simulink model "ofdm_txrx_mimo"  (Simulink 7.1)
settings: Solver=ode45  StartTime=0.0  StopTime=6e3  RelTol=1e-3
PreLoadFcn:
  | ofdm_rx_mimo_init
InitFcn:
  | ofdm_rx_mimo_init
StartFcn:
  | ofdm_rx_mimo_init
values from neighbouring files (symbols this model uses):
  BER_RAM_init_size = 2048    [ofdm_tx_mimo_init.m]
  CPLength = 16    [ofdm_tx_mimo_init.m]
  CRCPolynomial = hex2dec('04c11db7')    [ofdm_tx_mimo_init.m]
  freqOffset_track_filtCoef_i = hex2dec('2500')/2^32    [ofdm_rx_mimo_init.m]
  freqOffset_track_filtCoef_p = hex2dec('d0000')/2^32    [ofdm_rx_mimo_init.m]
  hi = zeros(1,length(cci))    [ofdm_rx_mimo_init.m]
  hr = zeros(1,length(ccr))    [ofdm_rx_mimo_init.m]
  max_CP_length = 16    [ofdm_tx_mimo_init.m]
  max_num_baseRateSymbols = 31    [ofdm_tx_mimo_init.m]
  max_num_subcarriers = 64    [ofdm_tx_mimo_init.m]
  max_num_trainingSymbols = 15    [ofdm_tx_mimo_init.m]
  max_numBytes = 16384    [ofdm_tx_mimo_init.m]
  max_OFDM_symbols = 2047    [ofdm_tx_mimo_init.m]
  modConstellation_qam16 = .75*[1 3 -1 -3]./3    [ofdm_tx_mimo_init.m]
  modConstellation_qam256 = 0.9375*[3 1 5 7 11 9 13 15 -3 -1 -5 -7 -11 -9 -13 -15]./15    [ofdm_tx_mimo_init.m]
  modConstellation_qam64 = 0.875*[3 1 5 7 -3 -1 -5 -7]./7    [ofdm_tx_mimo_init.m]
  modConstellation_qpsk = [1 -1]./sqrt(2)    [ofdm_tx_mimo_init.m]
  numBaseRateSymbols = 2    [ofdm_tx_mimo_init.m]
  numSubcarriers = 64    [ofdm_tx_mimo_init.m]
  numTrainingSymbols = 2    [ofdm_tx_mimo_init.m]
  pilot1_indicies = 7 + ( (64-7) * 2^16)    (= 3735559)    [ofdm_tx_mimo_init.m]
  pilot2_indicies = 21 + ( (64-21) * 2^16)    (= 2818069)    [ofdm_tx_mimo_init.m]
  pilotValue_neg = hex2dec('8000') + (2^16 * 0)    [ofdm_tx_mimo_init.m]
  pilotValue_pos = hex2dec('7FFF') + (2^16 * 0)    [ofdm_tx_mimo_init.m]
  pkt_crossCorr_thresh = 0.7    [ofdm_rx_mimo_init.m]
  pkt_energy_thresh = 0    [ofdm_rx_mimo_init.m]
  pkt_numPayloadBytes = 1400+42-6    (= 1436)    [ofdm_tx_mimo_init.m]
  pktByteNums = numHeaderBytes +  				byteIndex_numPayloadBytes(1)*2^8 +  				byteIndex_numPayloadBytes(2)*2^16 +  				byteIndex_simpleDynModMasks * 2^24    [ofdm_rx_mimo_init.m]
  PktDet_Delay = 26 + Symbol_Timing_Offset*2^7    (= 1050)    [ofdm_rx_mimo_init.m]
  preamble = 6*[0 shortsyms_10 longsyms_2]    [ofdm_tx_mimo_init.m]
  preamble_I = real(preamble)    [ofdm_tx_mimo_init.m]
  preamble_Q = imag(preamble)    [ofdm_tx_mimo_init.m]
  RAM_init_size = max_numBytes    (= 16384)    [ofdm_tx_mimo_init.m]
  reg_Interrupt_PktBuf_Control = reg_InterruptControl +  	1 * 2^16 +  	0 * 2^24    (= 65656)    [ofdm_rx_mimo_init.m]
  rx_controlBits = 1 * 2  		 + 1 * 4  		 + 0 * 8  		 + 1 * 16 * rx_SISO_Mode  		 + 1 * 32  		 + 1 * 64  		 + 0 * 128  		 + 1 * 256  		 + 0 * 512  		 + 1 * 1024  		 + 0 * 2048  		 + 1 * 4096  		 + 1 * 8192  		 + 0 * 16384  		 + 1 * 65536  		 + 1 * 131072  		 + 0 * 262144  		 + 1 * 524288    [ofdm_rx_mimo_init.m]
  Rx_PhaseNoiseTrack_K = 0.6094    [ofdm_rx_mimo_init.m]
  rx_postEq_scaling = round(rxScaling*2^11) * (1 + 2^16)    [ofdm_rx_mimo_init.m]
  RxDataScrambling_Seq = [40 198 78 63 82 173 102 245 48 111 172 115 147 230 216 93 72 65 62 2 205 242 122 90 128 83 105 97 73 10 5 252]    [ofdm_tx_mimo_init.m]
  simOnly_numSamples = length(preamble)+( (numSubcarriers+CPLength)*(numTrainingSymbols + numBaseRateSymbols + numFullRateSymbols/2) )    [ofdm_tx_mimo_init.m]
  train = [train train]    [ofdm_tx_mimo_init.m]
  tx_controlBits = (antB_preambleShift * 2^4) + (2^0 * tx_SISO_Mode) + 4*0 + 8*1    (= 73)    [ofdm_tx_mimo_init.m]
  TxDataScrambling_Seq = [40 198 78 63 82 173 102 245 48 111 172 115 147 230 216 93 72 65 62 2 205 242 122 90 128 83 105 97 73 10 5 252]    [ofdm_tx_mimo_init.m]
  txReg_symbolCounts = (2^16 * numFullRateSymbols) + (2^8 *numBaseRateSymbols ) + numTrainingSymbols    [ofdm_tx_mimo_init.m]
  TxRx_FFTScaling = bin2dec('010111') + (bin2dec('000101') * 2^6)    [ofdm_tx_mimo_init.m]
undefined symbols (not defined in the file or in its folder): AntA, AntB, BaseRate, CRC_table_gen, gcbh, intfilt, pktTiming_count_payloadStart, pktTiming_symbol_length, xlBlockLoadCallback, xlGetSimulinkPeriod
libraries: xbsIndex_r4 x4212, dspmlti4 x4, dspsigops x4, commchan3 x2, simulink x2, commrflib2 x2, dspsrcs4 x1
(part 15 of 43)

== system ofdm_txrx_mimo/OFDM Tx MIMO/TxControl/FFT Start Gen ==
  note: First two symbols are always training and should be processed immediately, back-to-back. The same IFFT
      | output will be repeated numTrainingSyms times. After this, the IFFT processes the base-rate symbols. Because
      | each base-rate symbol is transmitted from both antennas, the IFFT waits a full output symbol period before processing
      | the next symbol. After the base rate symbols, the multiplexing of full-rate symbols begins, so the IFFT is triggered twice
      | per output symbol period (computing two output symbols- one per antenna- per period).
  SymNum : Inport  OutDataType=sfix(16)  OutScaling=2^0
  AddSub1 : xbsIndex_r4/AddSub  mode=Addition  use_carryin=off  use_carryout=off  en=off  latency=0  precision=Full  arith_type=Unsigned  n_bits=10  bin_pt=0  quantization=Truncate  overflow=Wrap  dbl_ovrd=off  use_behavioral_HDL=on  pipelined=off  use_rpm=on  hw_selection=Fabric  block_type=addsub
      settings: xl_area=[0, 0, 0, 0, 0, 0, 0]  block_version=VER_STRING_GOES_HERE
  Constant9 : xbsIndex_r4/Constant  arith_type=Unsigned  const=1  n_bits=1  bin_pt=0  explicit_period=off  period=1  equ=P=C  opselect=C  inp2=PCIN>>17  opr=+  inp1=P  carry=CIN  dbl_ovrd=off  block_type=constant
      settings: block_version=VER_STRING_GOES_HERE
  Inverter : xbsIndex_r4/Inverter  en=off  latency=0  dbl_ovrd=off  block_type=inv
      settings: block_version=VER_STRING_GOES_HERE
  LSB : xbsIndex_r4/Slice  nbits=1  boolean_output=on  mode=Lower Bit Location + Width  bit1=0  base1=LSB of Input  bit0=0  base0=LSB of Input  dbl_ovrd=off  block_type=slice
      settings: infoedit="Extracts a given range of bits from each input sample and presents it at the output.  The output type is ordinarily unsigned with binary point at zero, but can be Boolean when the slice is one bit wide.<P><P>Hardware notes: In hardware this block costs nothing."  block_version=VER_STRING_GOES_HERE
  Logical : xbsIndex_r4/Logical  logical_function=OR  inputs=3  en=off  latency=0  precision=Full  arith_type=Unsigned  n_bits=16  bin_pt=0  align_bp=on  dbl_ovrd=off  block_type=logical
      settings: block_version=VER_STRING_GOES_HERE
  Logical1 : xbsIndex_r4/Logical  logical_function=AND  inputs=3  en=off  latency=0  precision=Full  arith_type=Unsigned  n_bits=16  bin_pt=0  align_bp=on  dbl_ovrd=off  block_type=logical
      settings: block_version=VER_STRING_GOES_HERE
  Output Scope2 : Scope  NumInputPorts=6
      settings: TimeRange=2200  YMin=0~0~0~-1~-1~-1  YMax=1~1~1~1~1~1  SaveName=ScopeData7
  Relational1 : xbsIndex_r4/Relational  mode=a>=b  en=off  latency=0  dbl_ovrd=off  block_type=relational
      settings: xl_area=[2 0 0 4 0 0 0]  block_version=VER_STRING_GOES_HERE
  Relational2 : xbsIndex_r4/Relational  mode=a<b  en=off  latency=0  dbl_ovrd=off  block_type=relational
      settings: xl_area=[2 0 0 4 0 0 0]  block_version=VER_STRING_GOES_HERE
  Relational3 : xbsIndex_r4/Relational  mode=a>=b  en=off  latency=0  dbl_ovrd=off  block_type=relational
      settings: xl_area=[2 0 0 4 0 0 0]  block_version=VER_STRING_GOES_HERE
  Relational9 : xbsIndex_r4/Relational  mode=a<=b  en=off  latency=0  dbl_ovrd=off  block_type=relational
      settings: xl_area=[2 0 0 4 0 0 0]  block_version=VER_STRING_GOES_HERE
  En : Outport  OutDataType=sfix(16)  OutScaling=2^0
  wires:
  Constant9/1 -> Relational9/2
  SymNum/1 -> Relational9/1, Relational1/1, Relational2/1, LSB/1, Relational3/1, Output Scope2/1
  Relational9/1 -> Logical/1, Output Scope2/2
  Logical/1 -> En/1, Output Scope2/5
  [reg_numTrainingSyms] -> Relational1/2, AddSub1/1
  Relational1/1 -> Logical1/1
  AddSub1/1 -> Relational2/2, Relational3/2
  [reg_numBaseRateSyms] -> AddSub1/2
  Relational2/1 -> Logical1/2
  LSB/1 -> Inverter/1
  Logical1/1 -> Logical/2, Output Scope2/3
  Relational3/1 -> Logical/3, Output Scope2/4
  Inverter/1 -> Logical1/3

== system ofdm_txrx_mimo/OFDM Tx MIMO/TxControl/Posedge1 ==
  D : Inport  OutDataType=sfix(16)  OutScaling=2^0
  Delay : xbsIndex_r4/Delay  en=off  latency=1  dbl_ovrd=off  reg_retiming=off  block_type=delay
      settings: infoedit=Hardware notes: A delay line is a chain, each link of which is an SRL16 followed by a flip-flop. If register retiming is enabled, the delay line is a chain of flip-flops.  xl_area=[1 1 0 0 0 0 0]  block_version=VER_STRING_GOES_HERE
  Inverter : xbsIndex_r4/Inverter  en=off  latency=0  dbl_ovrd=off  block_type=inv
      settings: xl_area=[0 0 0 0 0 0 0]  block_version=VER_STRING_GOES_HERE
  Logical : xbsIndex_r4/Logical  logical_function=AND  inputs=2  en=off  latency=0  precision=Full  arith_type=Unsigned  n_bits=8  bin_pt=2  align_bp=on  dbl_ovrd=off  block_type=logical
      settings: xl_area=[1 0 0 1 0 0 0]  block_version=VER_STRING_GOES_HERE
  Q : Outport  OutDataType=sfix(16)  OutScaling=2^0
  wires:
  Logical/1 -> Q/1
  D/1 -> Delay/1, Logical/1
  Inverter/1 -> Logical/2
  Delay/1 -> Inverter/1

== system ofdm_txrx_mimo/OFDM Tx MIMO/TxControl/Posedge2 ==
  D : Inport  OutDataType=sfix(16)  OutScaling=2^0
  Delay : xbsIndex_r4/Delay  en=off  latency=1  dbl_ovrd=off  reg_retiming=off  block_type=delay
      settings: infoedit=Hardware notes: A delay line is a chain, each link of which is an SRL16 followed by a flip-flop. If register retiming is enabled, the delay line is a chain of flip-flops.  xl_area=[1 1 0 0 0 0 0]  block_version=VER_STRING_GOES_HERE
  Inverter : xbsIndex_r4/Inverter  en=off  latency=0  dbl_ovrd=off  block_type=inv
      settings: xl_area=[0 0 0 0 0 0 0]  block_version=VER_STRING_GOES_HERE
  Logical : xbsIndex_r4/Logical  logical_function=AND  inputs=2  en=off  latency=0  precision=Full  arith_type=Unsigned  n_bits=1  bin_pt=0  align_bp=on  dbl_ovrd=off  block_type=logical
      settings: xl_area=[1 0 0 1 0 0 0]  block_version=VER_STRING_GOES_HERE
  Q : Outport  OutDataType=sfix(16)  OutScaling=2^0
  wires:
  Delay/1 -> Inverter/1
  Inverter/1 -> Logical/2
  D/1 -> Logical/1, Delay/1
  Logical/1 -> Q/1

== system ofdm_txrx_mimo/OFDM Tx MIMO/TxControl/Start/Done Control ==
  TxDone : Inport  OutDataType=sfix(16)  OutScaling=2^0
  Start : Inport  Port=2  OutDataType=sfix(16)  OutScaling=2^0
  Constant2 : xbsIndex_r4/Constant  arith_type=Boolean  const=1  n_bits=1  bin_pt=0  explicit_period=off  period=1  equ=P=C  opselect=C  inp2=PCIN>>17  opr=+  inp1=P  carry=CIN  dbl_ovrd=off  block_type=constant
      settings: block_version=VER_STRING_GOES_HERE
  Convert1 : xbsIndex_r4/Convert  arith_type=Boolean  n_bits=16  bin_pt=14  quantization=Truncate  overflow=Wrap  latency=0  dbl_ovrd=off  pipeline=off  block_type=convert
      settings: block_version=8.2.02
  Convert2 : xbsIndex_r4/Convert  arith_type=Boolean  n_bits=16  bin_pt=14  quantization=Truncate  overflow=Wrap  latency=0  dbl_ovrd=off  pipeline=off  block_type=convert
      settings: block_version=8.2.02
  Inverter : xbsIndex_r4/Inverter  en=off  latency=0  dbl_ovrd=off  block_type=inv
      settings: block_version=8.2.02
  Logical1 : xbsIndex_r4/Logical  logical_function=OR  inputs=2  en=off  latency=0  precision=Full  arith_type=Unsigned  n_bits=8  bin_pt=2  align_bp=on  dbl_ovrd=off  block_type=logical
      settings: xl_area=[1 0 0 1 0 0 0]  block_version=VER_STRING_GOES_HERE
  Logical4 : xbsIndex_r4/Logical  logical_function=AND  inputs=2  en=off  latency=0  precision=Full  arith_type=Unsigned  n_bits=8  bin_pt=2  align_bp=on  dbl_ovrd=off  block_type=logical
      settings: xl_area=[1 0 0 1 0 0 0]  block_version=VER_STRING_GOES_HERE
  Posedge2 : Subsystem
  Posedge3 : Subsystem
  S-R Latch : Subsystem
  Tx_PktDone : xbsIndex_r4/Gateway Out  hdl_port=on  timing_constraint=None  locs_specified=off  LOCs={}  block_type=gatewayout
      settings: infoedit="Gateway out block.  Converts Xilinx fixed point inputs into ouputs of type Simulink integer, double, or fixed point.<P><P>Hardware notes:  In hardware these blocks become top level output ports or are discarded, depending on how they are configured."  xl_area=[0 0 0 0 1 0 0]  block_version=VER_STRING_GOES_HERE
  Up Sample1 : xbsIndex_r4/Up Sample  sample_ratio=2  copy_samples=on  dbl_ovrd=off  block_type=usamp
      settings: infoedit="Up samples input data.  Inserted values  can be zeros or copies of the most recent input sample.<P><P>Hardware notes: No hardware is needed if inserted values are copies of the input sample; otherwise, a mux and single bit flip-flop are used."  block_version=VER_STRING_GOES_HERE
  Up Sample2 : xbsIndex_r4/Up Sample  sample_ratio=2  copy_samples=on  dbl_ovrd=off  block_type=usamp
      settings: infoedit="Up samples input data.  Inserted values  can be zeros or copies of the most recent input sample.<P><P>Hardware notes: No hardware is needed if inserted values are copies of the input sample; otherwise, a mux and single bit flip-flop are used."  block_version=VER_STRING_GOES_HERE
  TxStart : Outport  OutDataType=sfix(16)  OutScaling=2^0
  tag scope: regTx_PktRunning=global, regTx_PktRunningEn=global
  wires:
  Start/1 -> Posedge2/1(D)
  TxDone/1 -> Posedge3/1(D)
  Logical1/1 -> S-R Latch/2(R)
  [TxReset] -> Logical1/2
  S-R Latch/1(Q) -> Up Sample1/1, Inverter/1
  Constant2/1 -> [regTx_PktRunningEn]
  Up Sample1/1 -> [regTx_PktRunning]
  Inverter/1 -> Convert2/1
  Logical4/1 -> TxStart/1
  Posedge3/1(Q) -> Logical1/1, Up Sample2/1
  Convert1/1 -> Logical4/1
  Convert2/1 -> Logical4/2
  Posedge2/1(Q) -> S-R Latch/1(S), Convert1/1
  Up Sample2/1 -> Tx_PktDone/1

== system ofdm_txrx_mimo/OFDM Tx MIMO/TxStart/Copy_Downsample ==
  D : Inport  OutDataType=sfix(16)  OutScaling=2^0
  Delay : xbsIndex_r4/Delay  en=off  latency=1  dbl_ovrd=off  reg_retiming=off  block_type=delay
      settings: infoedit=Hardware notes: A delay line is a chain, each link of which is an SRL16 followed by a flip-flop. If register retiming is enabled, the delay line is a chain of flip-flops.  block_version=VER_STRING_GOES_HERE
  Down Sample : xbsIndex_r4/Down Sample  sample_ratio=2  sample_phase="Last Value of Frame  (most efficient)"  en=off  latency=1  dbl_ovrd=off  block_type=dsamp
      settings: block_version=VER_STRING_GOES_HERE
  Logical : xbsIndex_r4/Logical  logical_function=OR  inputs=2  en=off  latency=0  precision=Full  arith_type=Unsigned  n_bits=16  bin_pt=0  align_bp=on  dbl_ovrd=off  block_type=logical
      settings: block_version=VER_STRING_GOES_HERE
  Q : Outport  OutDataType=sfix(16)  OutScaling=2^0
  wires:
  Down Sample/1 -> Q/1
  Logical/1 -> Down Sample/1
  D/1 -> Logical/2, Delay/1
  Delay/1 -> Logical/1

== system ofdm_txrx_mimo/Memory-mapped Registers/TxRx Registers/InterruptControl/Posedge ==
  note: Rising Edge Detection
  D : Inport  OutDataType=sfix(16)  OutScaling=2^0
  Inverter : xbsIndex_r4/Inverter  en=off  latency=1  dbl_ovrd=off  block_type=inv
      settings: xl_area=[0 0 0 0 0 0 0]  block_version=VER_STRING_GOES_HERE
  Logical1 : xbsIndex_r4/Logical  logical_function=AND  inputs=2  en=off  latency=0  precision=Full  arith_type=Unsigned  n_bits=1  bin_pt=0  align_bp=on  dbl_ovrd=off  block_type=logical
      settings: xl_area=[1 0 0 1 0 0 0]  block_version=VER_STRING_GOES_HERE
  Q : Outport  OutDataType=sfix(16)  OutScaling=2^0
  wires:
  Inverter/1 -> Logical1/1
  Logical1/1 -> Q/1
  D/1 -> Logical1/2, Inverter/1

== system ofdm_txrx_mimo/Memory-mapped Registers/TxRx Registers/InterruptControl/Posedge1 ==
  note: Rising Edge Detection
  D : Inport  OutDataType=sfix(16)  OutScaling=2^0
  Inverter : xbsIndex_r4/Inverter  en=off  latency=1  dbl_ovrd=off  block_type=inv
      settings: xl_area=[0 0 0 0 0 0 0]  block_version=VER_STRING_GOES_HERE
  Logical1 : xbsIndex_r4/Logical  logical_function=AND  inputs=2  en=off  latency=0  precision=Full  arith_type=Unsigned  n_bits=1  bin_pt=0  align_bp=on  dbl_ovrd=off  block_type=logical
      settings: xl_area=[1 0 0 1 0 0 0]  block_version=VER_STRING_GOES_HERE
  Q : Outport  OutDataType=sfix(16)  OutScaling=2^0
  wires:
  D/1 -> Inverter/1, Logical1/2
  Logical1/1 -> Q/1
  Inverter/1 -> Logical1/1

== system ofdm_txrx_mimo/Memory-mapped Registers/TxRx Registers/InterruptControl/Posedge2 ==
  note: Rising Edge Detection
  D : Inport  OutDataType=sfix(16)  OutScaling=2^0
  Inverter : xbsIndex_r4/Inverter  en=off  latency=1  dbl_ovrd=off  block_type=inv
      settings: xl_area=[0 0 0 0 0 0 0]  block_version=VER_STRING_GOES_HERE
  Logical1 : xbsIndex_r4/Logical  logical_function=AND  inputs=2  en=off  latency=0  precision=Full  arith_type=Unsigned  n_bits=1  bin_pt=0  align_bp=on  dbl_ovrd=off  block_type=logical
      settings: xl_area=[1 0 0 1 0 0 0]  block_version=VER_STRING_GOES_HERE
  Q : Outport  OutDataType=sfix(16)  OutScaling=2^0
  wires:
  Inverter/1 -> Logical1/1
  Logical1/1 -> Q/1
  D/1 -> Logical1/2, Inverter/1

== system ofdm_txrx_mimo/OFDM Rx MIMO/ADC Inputs & Antenna Selection/Antenna Selection/Ant Sel Logic ==
  note: Two reasons to select antenna B:
      | 1) In SISO mode, switching diversity is enabled and antenna B's gains are lower (i.e. its SNR is higher)
      | 2) In SISO mode, switchinv diversity is disabled and user asserts noSwitch_forceAntB config bit
  G(B) < G(A) : Inport  OutDataType=sfix(16)  OutScaling=2^0
  B_Vin : Inport  Port=2  OutDataType=sfix(16)  OutScaling=2^0
  Down Sample1 : xbsIndex_r4/Down Sample  sample_ratio=2  sample_phase="Last Value of Frame  (most efficient)"  en=off  latency=1  dbl_ovrd=off  block_type=dsamp
      settings: block_version=8.2.02
  Inverter : xbsIndex_r4/Inverter  en=off  latency=0  dbl_ovrd=off  block_type=inv
      settings: block_version=8.2.02
  Logical1 : xbsIndex_r4/Logical  logical_function=AND  inputs=4  en=off  latency=0  precision=Full  arith_type=Unsigned  n_bits=8  bin_pt=2  align_bp=on  dbl_ovrd=off  block_type=logical
      settings: xl_area=[1 0 0 1 0 0 0]  block_version=VER_STRING_GOES_HERE
  Logical2 : xbsIndex_r4/Logical  logical_function=AND  inputs=3  en=off  latency=0  precision=Full  arith_type=Unsigned  n_bits=8  bin_pt=2  align_bp=on  dbl_ovrd=off  block_type=logical
      settings: xl_area=[1 0 0 1 0 0 0]  block_version=VER_STRING_GOES_HERE
  Logical3 : xbsIndex_r4/Logical  logical_function=OR  inputs=2  en=off  latency=0  precision=Full  arith_type=Unsigned  n_bits=8  bin_pt=2  align_bp=on  dbl_ovrd=off  block_type=logical
      settings: xl_area=[1 0 0 1 0 0 0]  block_version=VER_STRING_GOES_HERE
  AntSel : Outport  OutDataType=sfix(16)  OutScaling=2^0
  wires:
  [regRx_SISOmode] -> Down Sample1/1
  Logical1/1 -> Logical3/2
  B_Vin/1 -> Logical1/4
  G(B) < G(A)/1 -> Logical1/3
  Down Sample1/1 -> Logical1/1, Logical2/2
  [regRx_switchingDivEn] -> Logical1/2, Inverter/1
  Logical3/1 -> AntSel/1
  [regRx_noSwitch_forceAntB] -> Logical2/1
  Inverter/1 -> Logical2/3
  Logical2/1 -> Logical3/1

== system ofdm_txrx_mimo/OFDM Rx MIMO/ADC Inputs & Antenna Selection/Antenna Selection/AntA_Gains ==
  Rst : Inport  OutDataType=sfix(16)  OutScaling=2^0
  Concat : xbsIndex_r4/Concat  num_inputs=2  dbl_ovrd=off  block_type=concat
      settings: block_version=8.2.02
  Constant : Constant  OutDataType=sfix(16)  OutScaling=2^0
  Constant2 : Constant  Value=15  OutDataType=sfix(16)  OutScaling=2^0
  Constant4 : Constant  Value=0  OutDataType=sfix(16)  OutScaling=2^0
  Down Sample4 : xbsIndex_r4/Down Sample  sample_ratio=4  sample_phase="Last Value of Frame  (most efficient)"  en=off  latency=1  dbl_ovrd=off  block_type=dsamp
      settings: block_version=8.2.02
  Down Sample5 : xbsIndex_r4/Down Sample  sample_ratio=4  sample_phase="Last Value of Frame  (most efficient)"  en=off  latency=1  dbl_ovrd=off  block_type=dsamp
      settings: block_version=8.2.02
  Down Sample6 : xbsIndex_r4/Down Sample  sample_ratio=4  sample_phase="Last Value of Frame  (most efficient)"  en=off  latency=1  dbl_ovrd=off  block_type=dsamp
      settings: block_version=8.2.02
  Posedge : Subsystem
  Register : xbsIndex_r4/Register  init=0  rst=on  en=on  dbl_ovrd=off  block_type=register
      settings: block_version=8.2.02
  Rx_AntA_AGC_Done : xbsIndex_r4/Gateway In  arith_type=Unsigned  n_bits=1  bin_pt=0  quantization="Round  (unbiased: +/- Inf)"  overflow=Saturate  period=1  dbl_ovrd=off  timing_constraint=None  locs_specified=off  LOCs={}  block_type=gatewayin
      settings: infoedit="Gateway in block.  Converts inputs of type Simulink integer, double and fixed point to  Xilinx fixed point type.<P><P>Hardware notes:  In hardware these blocks become top level input ports."  block_version=VER_STRING_GOES_HERE
  Rx_AntA_GainBB : xbsIndex_r4/Gateway In  arith_type=Unsigned  n_bits=5  bin_pt=0  quantization="Round  (unbiased: +/- Inf)"  overflow=Saturate  period=1  dbl_ovrd=off  timing_constraint=None  locs_specified=off  LOCs={}  block_type=gatewayin
      settings: infoedit="Gateway in block.  Converts inputs of type Simulink integer, double and fixed point to  Xilinx fixed point type.<P><P>Hardware notes:  In hardware these blocks become top level input ports."  block_version=VER_STRING_GOES_HERE
  Rx_AntA_GainRF : xbsIndex_r4/Gateway In  arith_type=Unsigned  n_bits=2  bin_pt=0  quantization="Round  (unbiased: +/- Inf)"  overflow=Saturate  period=1  dbl_ovrd=off  timing_constraint=None  locs_specified=off  LOCs={}  block_type=gatewayin
      settings: infoedit="Gateway in block.  Converts inputs of type Simulink integer, double and fixed point to  Xilinx fixed point type.<P><P>Hardware notes:  In hardware these blocks become top level input ports."  block_version=VER_STRING_GOES_HERE
  S-R Latch : Subsystem
  Vout : Outport  OutDataType=sfix(16)  OutScaling=2^0
  GainVec : Outport  Port=2  OutDataType=sfix(16)  OutScaling=2^0
  wires:
  Constant/1 -> Rx_AntA_GainRF/1
  Constant2/1 -> Rx_AntA_GainBB/1
  Rx_AntA_GainRF/1 -> Down Sample4/1
  Rx_AntA_GainBB/1 -> Down Sample5/1
  Constant4/1 -> Rx_AntA_AGC_Done/1
  Rx_AntA_AGC_Done/1 -> Down Sample6/1
  Concat/1 -> Register/1
  Posedge/1(Q) -> Register/3, S-R Latch/1(S)
  Down Sample4/1 -> Concat/1
  Down Sample5/1 -> Concat/2
  Down Sample6/1 -> Posedge/1(D)
  Rst/1 -> Register/2, S-R Latch/2(R)
  S-R Latch/1(Q) -> Vout/1
  Register/1 -> GainVec/1

== system ofdm_txrx_mimo/OFDM Rx MIMO/ADC Inputs & Antenna Selection/Antenna Selection/AntB_Gains ==
  Rst : Inport  OutDataType=sfix(16)  OutScaling=2^0
  Concat1 : xbsIndex_r4/Concat  num_inputs=2  dbl_ovrd=off  block_type=concat
      settings: block_version=8.2.02
  Constant1 : Constant  OutDataType=sfix(16)  OutScaling=2^0
  Constant3 : Constant  Value=17  OutDataType=sfix(16)  OutScaling=2^0
  Constant5 : Constant  Value=0  OutDataType=sfix(16)  OutScaling=2^0
  Down Sample7 : xbsIndex_r4/Down Sample  sample_ratio=4  sample_phase="Last Value of Frame  (most efficient)"  en=off  latency=1  dbl_ovrd=off  block_type=dsamp
      settings: block_version=8.2.02
  Down Sample8 : xbsIndex_r4/Down Sample  sample_ratio=4  sample_phase="Last Value of Frame  (most efficient)"  en=off  latency=1  dbl_ovrd=off  block_type=dsamp
      settings: block_version=8.2.02
  Down Sample9 : xbsIndex_r4/Down Sample  sample_ratio=4  sample_phase="Last Value of Frame  (most efficient)"  en=off  latency=1  dbl_ovrd=off  block_type=dsamp
      settings: block_version=8.2.02
  Posedge1 : Subsystem
  Register1 : xbsIndex_r4/Register  init=0  rst=on  en=on  dbl_ovrd=off  block_type=register
      settings: block_version=8.2.02
  Rx_AntB_AGC_Done : xbsIndex_r4/Gateway In  arith_type=Unsigned  n_bits=1  bin_pt=0  quantization="Round  (unbiased: +/- Inf)"  overflow=Saturate  period=1  dbl_ovrd=off  timing_constraint=None  locs_specified=off  LOCs={}  block_type=gatewayin
      settings: infoedit="Gateway in block.  Converts inputs of type Simulink integer, double and fixed point to  Xilinx fixed point type.<P><P>Hardware notes:  In hardware these blocks become top level input ports."  block_version=VER_STRING_GOES_HERE
  Rx_AntB_GainBB : xbsIndex_r4/Gateway In  arith_type=Unsigned  n_bits=5  bin_pt=0  quantization="Round  (unbiased: +/- Inf)"  overflow=Saturate  period=1  dbl_ovrd=off  timing_constraint=None  locs_specified=off  LOCs={}  block_type=gatewayin
      settings: infoedit="Gateway in block.  Converts inputs of type Simulink integer, double and fixed point to  Xilinx fixed point type.<P><P>Hardware notes:  In hardware these blocks become top level input ports."  block_version=VER_STRING_GOES_HERE
  Rx_AntB_GainRF : xbsIndex_r4/Gateway In  arith_type=Unsigned  n_bits=2  bin_pt=0  quantization="Round  (unbiased: +/- Inf)"  overflow=Saturate  period=1  dbl_ovrd=off  timing_constraint=None  locs_specified=off  LOCs={}  block_type=gatewayin
      settings: infoedit="Gateway in block.  Converts inputs of type Simulink integer, double and fixed point to  Xilinx fixed point type.<P><P>Hardware notes:  In hardware these blocks become top level input ports."  block_version=VER_STRING_GOES_HERE
  S-R Latch1 : Subsystem
  Vout : Outport  OutDataType=sfix(16)  OutScaling=2^0
  GainVec : Outport  Port=2  OutDataType=sfix(16)  OutScaling=2^0
  wires:
  Constant1/1 -> Rx_AntB_GainRF/1
  Constant3/1 -> Rx_AntB_GainBB/1
  Rx_AntB_GainRF/1 -> Down Sample7/1
  Rx_AntB_GainBB/1 -> Down Sample8/1
  Constant5/1 -> Rx_AntB_AGC_Done/1
  Rx_AntB_AGC_Done/1 -> Down Sample9/1
  Concat1/1 -> Register1/1
  Posedge1/1(Q) -> Register1/3, S-R Latch1/1(S)
  Down Sample7/1 -> Concat1/1
  Down Sample8/1 -> Concat1/2
  Down Sample9/1 -> Posedge1/1(D)
  Rst/1 -> Register1/2, S-R Latch1/2(R)
  S-R Latch1/1(Q) -> Vout/1
  Register1/1 -> GainVec/1

== system ofdm_txrx_mimo/OFDM Rx MIMO/ADC Inputs & Antenna Selection/Antenna Selection/Posedge2 ==
  note: Rising Edge Detection
  D : Inport  OutDataType=sfix(16)  OutScaling=2^0
  Delay : xbsIndex_r4/Delay  en=off  latency=1  dbl_ovrd=off  reg_retiming=off  block_type=delay
      settings: infoedit=Hardware notes: A delay line is a chain, each link of which is an SRL16 followed by a flip-flop. If register retiming is enabled, the delay line is a chain of flip-flops.  xl_area=[1 1 0 0 0 0 0]  block_version=VER_STRING_GOES_HERE
  Inverter : xbsIndex_r4/Inverter  en=off  latency=0  dbl_ovrd=off  block_type=inv
      settings: xl_area=[0 0 0 0 0 0 0]  block_version=VER_STRING_GOES_HERE
  Logical1 : xbsIndex_r4/Logical  logical_function=AND  inputs=2  en=off  latency=0  precision=Full  arith_type=Unsigned  n_bits=8  bin_pt=2  align_bp=on  dbl_ovrd=off  block_type=logical
      settings: xl_area=[1 0 0 1 0 0 0]  block_version=VER_STRING_GOES_HERE
  ToBool : xbsIndex_r4/Convert  arith_type=Boolean  n_bits=16  bin_pt=14  quantization=Truncate  overflow=Wrap  latency=0  dbl_ovrd=off  pipeline=off  block_type=convert
      settings: block_version=8.2.02
  Q : Outport  OutDataType=sfix(16)  OutScaling=2^0
  wires:
  ToBool/1 -> Q/1
  Delay/1 -> Logical1/1
  Inverter/1 -> Delay/1
  D/1 -> Inverter/1, Logical1/2
  Logical1/1 -> ToBool/1

== system ofdm_txrx_mimo/OFDM Rx MIMO/Coarse Freq Correction/Coarse Freq Offset Calc Combo/Accum Control ==
  En : Inport  OutDataType=sfix(16)  OutScaling=2^0
  Reset : Inport  Port=2  OutDataType=sfix(16)  OutScaling=2^0
  8xDebounce : Subsystem
  Addressable Shift Register : xbsIndex_r4/Addressable Shift Register  infer_latency=on  depth=2  initVector=[0]  en=off  dbl_ovrd=off  use_behavioral_HDL=off  use_rpm=off  block_type=addrsr
      settings: block_version=8.2.02
      settings: infoedit=|
      | Delay of  configurable length.  Any element  in the delay
      | 
      |     line   can    be   addressed   and   driven    onto   the   output
      | 
      |     port.<P><P>Hardware  notes: Implemented  using SRL16s.
      | 
      |     If Virtex-4, Virtex-II or Spartan-3 devices are used, multiple SRLC16s are cascaded together.
  Coarse Freq Scope2 : Scope  NumInputPorts=5
      settings: TimeRange=1600  YMin=-1~-1~-1~-1~0.95  YMax=1~1~1~1~1.05  SaveName=ScopeData63
  Delay4 : xbsIndex_r4/Delay  en=off  latency=64+8  dbl_ovrd=off  reg_retiming=off  block_type=delay
      settings: infoedit=Hardware notes: A delay line is a chain, each link of which is an SRL16 followed by a flip-flop. If register retiming is enabled, the delay line is a chain of flip-flops.  xl_area=[1 1 0 0 0 0 0]  block_version=VER_STRING_GOES_HERE
  Display : Display
  Gateway Out{1..2,10..11,13..14} (6 alike) : xbsIndex_r4/Gateway Out  hdl_port=off  timing_constraint=None  locs_specified=off  LOCs={}  block_type=gatewayout
      settings: infoedit="Gateway out block.  Converts Xilinx fixed point inputs into ouputs of type Simulink integer, double, or fixed point.<P><P>Hardware notes:  In hardware these blocks become top level output ports or are discarded, depending on how they are configured."  xl_area=[0, 0, 0, 0, 0, 0, 0]  block_version=VER_STRING_GOES_HERE
  Inverter1 : xbsIndex_r4/Inverter  en=off  latency=0  dbl_ovrd=off  block_type=inv
      settings: xl_area=[0 0 0 0 0 0 0]  block_version=VER_STRING_GOES_HERE
  Logical1 : xbsIndex_r4/Logical  logical_function=AND  inputs=2  en=off  latency=0  precision=Full  arith_type=Unsigned  n_bits=8  bin_pt=2  align_bp=on  dbl_ovrd=off  block_type=logical
      settings: xl_area=[0, 0, 0, 0, 0, 0, 0]  block_version=VER_STRING_GOES_HERE
  Logical2 : xbsIndex_r4/Logical  logical_function=AND  inputs=2  en=off  latency=0  precision=Full  arith_type=Unsigned  n_bits=8  bin_pt=2  align_bp=on  dbl_ovrd=off  block_type=logical
      settings: xl_area=[0, 0, 0, 0, 0, 0, 0]  block_version=VER_STRING_GOES_HERE
  Posedge3 : Subsystem
  S-R Latch1 : Subsystem
  " En" : Outport  OutDataType=sfix(16)  OutScaling=2^0
  wires:
  Logical1/1 -> " En"/1, Gateway Out13/1
  En/1 -> Gateway Out11/1, Logical2/1
  Gateway Out2/1 -> Display/1
  [regRx_CFOCalc_Dly] -> Gateway Out2/1, Addressable Shift Register/2
  Addressable Shift Register/1 -> 8xDebounce/1(D)
  Posedge3/1(Q) -> Gateway Out1/1, Logical2/2
  8xDebounce/1(Q) -> Posedge3/1(D)
  [longCorrAboveThresh] -> Addressable Shift Register/1, Gateway Out10/1
  Reset/1 -> S-R Latch1/1(R), Gateway Out14/1
  Gateway Out10/1 -> Coarse Freq Scope2/1
  Gateway Out11/1 -> Coarse Freq Scope2/2
  Gateway Out13/1 -> Coarse Freq Scope2/4
  Gateway Out14/1 -> Coarse Freq Scope2/5
  Gateway Out1/1 -> Coarse Freq Scope2/3
  Logical2/1 -> S-R Latch1/2(S)
  S-R Latch1/1(Q) -> Logical1/1, Delay4/1
  Delay4/1 -> Inverter1/1
  Inverter1/1 -> Logical1/2

== system ofdm_txrx_mimo/OFDM Rx MIMO/Coarse Freq Correction/Coarse Freq Offset Calc Combo/Arctan2 ==
  I : Inport  OutDataType=sfix(16)  OutScaling=2^0
  Q : Inport  Port=2  OutDataType=sfix(16)  OutScaling=2^0
  CORDIC ATAN1 : Subsystem  mask(Xilinx CORDIC ATAN){stages=16, pe_nbits=16, pe_binpt=13, pipeline_x=repmat([1 0],1,8), prev_stages=16, pipeline=[1  0  1  0  1  0  1  0  1  0  1  0  1  0  1  0]}
      mask prompts: stages "Number of Processing Elements (integer value starting from 1)"; pe_nbits "X, Y Data Width"; pe_binpt "X, Y Binary Point Position"; pipeline_x "Latency for each Processing Element [1 0 0 1]"; prev_stages "Previous Number of Stages"; pipeline "Previous Pipeline Values"
      mask help: A parallel implementation, circular vectoring mode CORDIC processor for performing rectangular-to-polar conversion. The number of iteration stages (and resultant output accuracy) and the processor arithmetic precision are controlled by the mask customization.
      mask init:
      | [bg,fg] = xlcmap('XBlock',0);
      | iPos = get_param(gcb,'Position');
      | iWidth=iPos(3)-iPos(1); iHeight=iPos(4)-iPos(2);
      | iCx=iWidth/2;
      | iCy=iHeight/2;
      | [logoX, logoY] = xlogo(iPos);
      | sav_gcb=gcb;
      | stages = round(sum(abs(stages)));
      | if (stages == 0)
      | 	stages = prev_stages;
      | end
      | x = zeros(1,stages);
      | pipe_size = max(size(pipeline_x));
      | if pipe_size >= stages
      | 	x(1,1:stages) = pipeline_x(1,1:stages);
      | else
      | 	x(1,1:pipe_size) = pipeline_x(1,1:pipe_size);
      | end
      | 
      | if (stages ~=prev_stages)
      | 	pipeline=x;
      | 	cordic_pe = find_system(gcb, 'lookUnderMasks', 'all', 'FollowLinks','on','masktype', 'CORDIC parallel PE');
      |     
      |   a=find_system(cordic_pe{1}, 'lookUnderMasks', 'all', 'FollowLinks','on','masktype', 'CORDIC iteration PE');
      |   for i= 2:length(a)
      |     for j=1:3
      | 	  delete_line(cordic_pe{1}, ['CORDIC PE' int2str(i-1) '/' int2str(j)], ['CORDIC PE' int2str(i) '/' int2str(j)]);
      |     end
      |   end
      |     if length(a)>0,
      |       delete_line(cordic_pe{1}, 'x/1', 'CORDIC PE1/1');
      |       delete_line(cordic_pe{1}, 'y/1', 'CORDIC PE1/2');
      |       delete_line(cordic_pe{1}, 'z/1', 'CORDIC PE1/3');
      |       delete_line(cordic_pe{1}, ['CORDIC PE' int2str(length(a)) '/1'],'X/1');
      |       delete_line(cordic_pe{1}, ['CORDIC PE' int2str(length(a)) '/2'],'Terminator/1');
      |       delete_line(cordic_pe{1}, ['CORDIC PE' int2str(length(a)) '/3'],'Z/1');
      |     elseif length(a)==0
      |       temp_port=get_param([cordic_pe{1} '/x'],'porthandles');
      |       temp_line=get_param(temp_port.Outport,'line');
      |       if temp_line>=0  delete_line(temp_line); end
      |       temp_port=get_param([cordic_pe{1} '/y'],'porthandles');
      |       temp_line=get_param(temp_port.Outport,'line');
      |       if temp_line>=0  delete_line(temp_line); end
      |       temp_port=get_param([cordic_pe{1} '/z'],'porthandles');
      |       temp_line=get_param(temp_port.Outport,'line');
      |       if temp_line>=0  delete_line(temp_line); end
      |     end
      | 
      |     for i=2:length(a)
      |       delete_block(a{i});
      |     end
      |     for i=2:stages,
      |         add_block([cordic_pe{1} '/CORDIC PE1'], [cordic_pe{1} '/CORDIC PE' int2str(i)], 'ii', int2str(i-1),'pe_nbits', 'pe_nbits','pe_binpt', 'pe_binpt','pipeline',['pipeline(1,' int2str(i) ')'],'position',[150+(i-1)*125, 70, 205+(i-1)*125, 130]);
      |     end
      | 
      |     for i= 2:stages,
      |       for j=1:3
      | 		add_line(cordic_pe{1}, ['CORDIC PE' int2str(i-1) '/' int2str(j)], ['CORDIC PE' int2str(i) '/' int2str(j)], 'autorouting', 'on');
      |       end
      |     end
      | 
      |     if stages==0
      |       add_line(cordic_pe{1}, 'x/1', 'X/1', 'autorouting', 'on');
      |       add_line(cordic_pe{1}, 'y/1', 'Terminator/1', 'autorouting', 'on');
      |       add_line(cordic_pe{1}, 'z/1', 'Z/1', 'autorouting', 'on');
      |     elseif stages>0
      |       add_line(cordic_pe{1}, 'x/1', 'CORDIC PE1/1', 'autorouting', 'on');
      |       add_line(cordic_pe{1}, 'y/1', 'CORDIC PE1/2', 'autorouting', 'on');
      |       add_line(cordic_pe{1}, 'z/1', 'CORDIC PE1/3', 'autorouting', 'on');
      | 
      |       set_param([cordic_pe{1} '/' 'X'], 'Position', [150+stages*125, 72, 180+stages*125, 86]);
      |       set_param([cordic_pe{1} '/' 'Terminator'], 'Position', [210+stages*125, 92, 225+stages*125, 108]);
      |       set_param([cordic_pe{1} '/' 'Z'], 'Position', [240+stages*125, 113, 270+stages*125, 127]);
      | 
      |       add_line(cordic_pe{1}, ['CORDIC PE' int2str(stages) '/1'], 'X/1', 'autorouting', 'on');
      |       add_line(cordic_pe{1}, ['CORDIC PE' int2str(stages) '/2'], 'Terminator/1', 'autorouting', 'on');
      |       add_line(cordic_pe{1}, ['CORDIC PE' int2str(stages) '/3'],'Z/1', 'autorouting', 'on');
      | 
      |       % force a "compile" of the constituent constant blocks to
      |       % workaround a data propagation problem
      |       find_system(cordic_pe{1}, 'lookUnderMasks', 'all', 'FollowLinks', 'on', 'MaskType','Xilinx Constant Block');
      |   end
      | 	set_param(sav_gcb, 'prev_stages', int2str(stages));
      | 	set_param(sav_gcb, 'pipeline', [ '[' int2str(x) ']']);
      | else
      | 	if (sum(pipeline~=x))
      | 		set_param(gcb, 'pipeline', [ '[' int2str(x) ']']);
      | 	end
      | end
      | str = sprintf('-%d',sum(x) + 3);
      settings: CopyFcn=set_param(gcb, 'MaskSelfModifiable', 'on', 'LinkStatus', 'none');
      mask Help: eval('xlWeb(xlhtmldoclink(''CORDIC ATAN''))');
      mask Display:
      | patch([0 iWidth iWidth 0],[0 0 iHeight iHeight] , bg);
      | patch(logoX,logoY , fg);
      | text(iCx-6,iCy,'z');
      | text(iCx,iCy+4,str);
      | port_label('input',1,'x');
      | port_label('input',2,'y');
      | port_label('output',1,'mag');
      | port_label('output',2,'atan');
      | plot([0 0 iWidth iWidth 0], [0 iHeight iHeight 0 0]);
  Down Sample : xbsIndex_r4/Down Sample  sample_ratio=4  sample_phase="Last Value of Frame  (most efficient)"  en=off  latency=1  dbl_ovrd=off  block_type=dsamp
      settings: block_version=8.2.02
  Up Sample : xbsIndex_r4/Up Sample  sample_ratio=4  copy_samples=on  dbl_ovrd=off  block_type=usamp
      settings: infoedit="Up samples input data.  Inserted values  can be zeros or copies of the most recent input sample.<P><P>Hardware notes: No hardware is needed if inserted values are copies of the input sample; otherwise, a mux and single bit flip-flop are used."  block_version=8.2.02
  Up Sample1 : xbsIndex_r4/Up Sample  sample_ratio=4  copy_samples=on  dbl_ovrd=off  block_type=usamp
      settings: infoedit="Up samples input data.  Inserted values  can be zeros or copies of the most recent input sample.<P><P>Hardware notes: No hardware is needed if inserted values are copies of the input sample; otherwise, a mux and single bit flip-flop are used."  block_version=8.2.02
  atan : Outport  OutDataType=sfix(16)  OutScaling=2^0
  wires:
  Down Sample/1 -> atan/1
  Q/1 -> Up Sample1/1
  I/1 -> Up Sample/1
  CORDIC ATAN1/2(Z) -> Down Sample/1
  Up Sample1/1 -> CORDIC ATAN1/2(y)
  Up Sample/1 -> CORDIC ATAN1/1(x)

== system ofdm_txrx_mimo/OFDM Rx MIMO/Coarse Freq Correction/Coarse Freq Offset Calc Combo/PhaseDiff ==
  p : Inport  OutDataType=sfix(16)  OutScaling=2^0
  AddSub1 : xbsIndex_r4/AddSub  mode=Subtraction  use_carryin=off  use_carryout=off  en=off  latency=0  precision=Full  arith_type="Signed  (2's comp)"  n_bits=32  bin_pt=30  quantization=Truncate  overflow=Wrap  dbl_ovrd=off  use_behavioral_HDL=off  pipelined=off  use_rpm=on  hw_selection=Fabric  block_type=addsub
      settings: block_version=VER_STRING_GOES_HERE
  Delay3 : xbsIndex_r4/Delay  en=off  latency=64  dbl_ovrd=off  reg_retiming=off  block_type=delay
      settings: infoedit=Hardware notes: A delay line is a chain, each link of which is an SRL16 followed by a flip-flop. If register retiming is enabled, the delay line is a chain of flip-flops.  xl_area=[0, 0, 0, 0, 0, 0, 0]  block_version=VER_STRING_GOES_HERE
  Unwrap : Subsystem
  diff(p0,p63) : Outport  OutDataType=sfix(16)  OutScaling=2^0
  wires:
  p/1 -> AddSub1/1, Delay3/1
  AddSub1/1 -> Unwrap/1(p)
  Delay3/1 -> AddSub1/2
  Unwrap/1(mod(p,2pi)) -> diff(p0,p63)/1

== system ofdm_txrx_mimo/OFDM Rx MIMO/Coarse Freq Correction/Coarse Freq Offset Calc Combo/Posedge ==
  D : Inport  OutDataType=sfix(16)  OutScaling=2^0
  Delay : xbsIndex_r4/Delay  en=off  latency=1  dbl_ovrd=off  reg_retiming=off  block_type=delay
      settings: infoedit=Hardware notes: A delay line is a chain, each link of which is an SRL16 followed by a flip-flop. If register retiming is enabled, the delay line is a chain of flip-flops.  xl_area=[1 1 0 0 0 0 0]  block_version=VER_STRING_GOES_HERE
  Inverter : xbsIndex_r4/Inverter  en=off  latency=0  dbl_ovrd=off  block_type=inv
      settings: xl_area=[0 0 0 0 0 0 0]  block_version=VER_STRING_GOES_HERE
  Logical1 : xbsIndex_r4/Logical  logical_function=AND  inputs=2  en=off  latency=0  precision=Full  arith_type=Unsigned  n_bits=8  bin_pt=2  align_bp=on  dbl_ovrd=off  block_type=logical
      settings: xl_area=[1 0 0 1 0 0 0]  block_version=VER_STRING_GOES_HERE
  Q : Outport  OutDataType=sfix(16)  OutScaling=2^0
  wires:
  D/1 -> Logical1/2, Delay/1
  Logical1/1 -> Q/1
  Inverter/1 -> Logical1/1
  Delay/1 -> Inverter/1

== system ofdm_txrx_mimo/OFDM Rx MIMO/Equalizer & Packetizer/Equalizer/Bus Selector ==
  H-I : Inport  OutDataType=sfix(16)  OutScaling=2^0
  H-Q : Inport  Port=2  OutDataType=sfix(16)  OutScaling=2^0
  Bus Selector : BusSelector  OutputSignals=signal1,signal2,signal3,signal4
  Bus Selector1 : BusSelector  OutputSignals=signal1,signal2,signal3,signal4
  Constant : xbsIndex_r4/Constant  arith_type=Signed (2's comp)  const=1/2  n_bits=18  bin_pt=16  explicit_period=off  period=1  equ=P=C  opselect=C  inp2=PCIN>>17  opr=+  inp1=P  carry=CIN  dbl_ovrd=off  block_type=constant
      settings: block_version=VER_STRING_GOES_HERE
  Mux : xbsIndex_r4/Mux  inputs=2  en=off  latency=0  precision=Full  arith_type=Unsigned  n_bits=16  bin_pt=14  quantization=Truncate  overflow=Wrap  dbl_ovrd=off  block_type=mux
      settings: block_version=VER_STRING_GOES_HERE
  Mux1 : xbsIndex_r4/Mux  inputs=2  en=off  latency=0  precision=Full  arith_type=Unsigned  n_bits=16  bin_pt=14  quantization=Truncate  overflow=Wrap  dbl_ovrd=off  block_type=mux
      settings: block_version=VER_STRING_GOES_HERE
  AA_I : Outport  OutDataType=sfix(16)  OutScaling=2^0
  AA_Q : Outport  Port=2  OutDataType=sfix(16)  OutScaling=2^0
  BB_I : Outport  Port=3  OutDataType=sfix(16)  OutScaling=2^0
  BB_Q : Outport  Port=4  OutDataType=sfix(16)  OutScaling=2^0
  AB_I : Outport  Port=5  OutDataType=sfix(16)  OutScaling=2^0
  AB_Q : Outport  Port=6  OutDataType=sfix(16)  OutScaling=2^0
  BA_I : Outport  Port=7  OutDataType=sfix(16)  OutScaling=2^0
  BA_Q : Outport  Port=8  OutDataType=sfix(16)  OutScaling=2^0
  wires:
  Bus Selector/1 -> AA_I/1  "<signal1>"
  Bus Selector/4 -> Mux1/2  "<signal4>"
  H-I/1 -> Bus Selector/1
  H-Q/1 -> Bus Selector1/1
  Bus Selector/3 -> BA_I/1  "<signal3>"
  Bus Selector/2 -> AB_I/1  "<signal2>"
  Bus Selector1/1 -> AA_Q/1  "<signal1>"
  Bus Selector1/2 -> AB_Q/1  "<signal2>"
  Bus Selector1/3 -> BA_Q/1  "<signal3>"
  Bus Selector1/4 -> Mux/2  "<signal4>"
  Constant/1 -> Mux/3, Mux1/3
  [regRx_SISOmode] -> Mux/1, Mux1/1
  Mux/1 -> BB_Q/1
  Mux1/1 -> BB_I/1

== system ofdm_txrx_mimo/OFDM Rx MIMO/Equalizer & Packetizer/Equalizer/Bus Selector1 ==
  Y_I : Inport  OutDataType=sfix(16)  OutScaling=2^0
  Y_Q : Inport  Port=2  OutDataType=sfix(16)  OutScaling=2^0
  Bus Selector1 : BusSelector  OutputSignals=signal1,signal2
  Bus Selector2 : BusSelector  OutputSignals=signal1,signal2
  YA_I : Outport  OutDataType=sfix(16)  OutScaling=2^0
  YA_Q : Outport  Port=2  OutDataType=sfix(16)  OutScaling=2^0
  YB_I : Outport  Port=3  OutDataType=sfix(16)  OutScaling=2^0
  YB_Q : Outport  Port=4  OutDataType=sfix(16)  OutScaling=2^0
  wires:
  Bus Selector1/1 -> YB_I/1  "<signal1>"
  Y_I/1 -> Bus Selector1/1
  Bus Selector1/2 -> YA_I/1  "<signal2>"
  Bus Selector2/1 -> YB_Q/1  "<signal1>"
  Y_Q/1 -> Bus Selector2/1
  Bus Selector2/2 -> YA_Q/1  "<signal2>"

== system ofdm_txrx_mimo/OFDM Rx MIMO/Equalizer & Packetizer/Equalizer/Complex Divider ==
  Re[A] : Inport  OutDataType=sfix(16)  OutScaling=2^0
  Im[A] : Inport  Port=2  OutDataType=sfix(16)  OutScaling=2^0
  Re[B] : Inport  Port=3  OutDataType=sfix(16)  OutScaling=2^0
  Im[B] : Inport  Port=4  OutDataType=sfix(16)  OutScaling=2^0
  Complex Divider Guts : Scope  NumInputPorts=8
      settings: TimeRange=6000  YMin=-0.75~-0.75~-1~-1.25~-0.75~-0.6~-0.5~-0.4  YMax=0.75~0.75~1.25~1~0.75~0.5~0.6~0.4  SaveName=ScopeData62
  ComplexConjMult_x2 : Subsystem
  Convert : xbsIndex_r4/Convert  arith_type="Signed  (2's comp)"  n_bits=17  bin_pt=15  quantization=Truncate  overflow=Saturate  latency=0  dbl_ovrd=off  pipeline=off  block_type=convert
      settings: block_version=VER_STRING_GOES_HERE
  Convert2 : xbsIndex_r4/Convert  arith_type="Signed  (2's comp)"  n_bits=17  bin_pt=15  quantization=Truncate  overflow=Saturate  latency=0  dbl_ovrd=off  pipeline=off  block_type=convert
      settings: block_version=VER_STRING_GOES_HERE
  Delay1 : xbsIndex_r4/Delay  en=off  latency=1  dbl_ovrd=off  reg_retiming=off  block_type=delay
      settings: infoedit=Hardware notes: A delay line is a chain, each link of which is an SRL16 followed by a flip-flop. If register retiming is enabled, the delay line is a chain of flip-flops.  xl_area=[0, 0, 0, 0, 0, 0, 0]  block_version=VER_STRING_GOES_HERE
  Delay2 : xbsIndex_r4/Delay  en=off  latency=1  dbl_ovrd=off  reg_retiming=off  block_type=delay
      settings: infoedit=Hardware notes: A delay line is a chain, each link of which is an SRL16 followed by a flip-flop. If register retiming is enabled, the delay line is a chain of flip-flops.  xl_area=[0, 0, 0, 0, 0, 0, 0]  block_version=VER_STRING_GOES_HERE
  Division : Subsystem
  done{1..7,12} (8 alike) : xbsIndex_r4/Gateway Out  hdl_port=off  timing_constraint=None  locs_specified=off  LOCs={}  block_type=gatewayout
      settings: infoedit="Gateway out block.  Converts Xilinx fixed point inputs into ouputs of type Simulink integer, double, or fixed point.<P><P>Hardware notes:  In hardware these blocks become top level output ports or are discarded, depending on how they are configured."  xl_area=[0 0 0 0 1 0 0]  block_version=VER_STRING_GOES_HERE
  Re[A/B] : Outport  OutDataType=sfix(16)  OutScaling=2^0
  Im[A/B] : Outport  Port=2  OutDataType=sfix(16)  OutScaling=2^0
  wires:
  Delay2/1 -> Division/4(Im[B])
  Delay1/1 -> Division/3(Re[B])
  Im[B]/1 -> Delay2/1, ComplexConjMult_x2/4(Im[B]), done1/1
  Re[B]/1 -> Delay1/1, ComplexConjMult_x2/3(Re[B]), done4/1
  Im[A]/1 -> ComplexConjMult_x2/2(Im[A]), done12/1
  Re[A]/1 -> ComplexConjMult_x2/1(Re[A]), done5/1
  ComplexConjMult_x2/2(Im1) -> Division/2(Im[CP]), done2/1
  ComplexConjMult_x2/1(Re) -> Division/1(Re[CP]), done3/1
  Convert/1 -> Re[A/B]/1, done7/1
  Convert2/1 -> Im[A/B]/1, done6/1
  done3/1 -> Complex Divider Guts/5
  done5/1 -> Complex Divider Guts/1
  done12/1 -> Complex Divider Guts/2
  done4/1 -> Complex Divider Guts/3
  done1/1 -> Complex Divider Guts/4
  done2/1 -> Complex Divider Guts/6
  Division/1(Re[A/B]) -> Convert/1
  Division/2(Im[A/B]) -> Convert2/1
  done7/1 -> Complex Divider Guts/7
  done6/1 -> Complex Divider Guts/8

== system ofdm_txrx_mimo/OFDM Rx MIMO/Equalizer & Packetizer/Equalizer/Determinant ==
  note: Calculates the determinant of the 2x2 matrix:
      | det([a b; c d]) = ad - bc
      | where a,b,c,d are all complex
  Re[a] : Inport  OutDataType=sfix(16)  OutScaling=2^0
  Im[a] : Inport  Port=2  OutDataType=sfix(16)  OutScaling=2^0
  Re[d] : Inport  Port=3  OutDataType=sfix(16)  OutScaling=2^0
  Im[d] : Inport  Port=4  OutDataType=sfix(16)  OutScaling=2^0
  Re[b] : Inport  Port=5  OutDataType=sfix(16)  OutScaling=2^0
  Im[b] : Inport  Port=6  OutDataType=sfix(16)  OutScaling=2^0
  Re[c] : Inport  Port=7  OutDataType=sfix(16)  OutScaling=2^0
  Im[c] : Inport  Port=8  OutDataType=sfix(16)  OutScaling=2^0
  Add1 : xbsIndex_r4/AddSub  mode=Subtraction  use_carryin=off  use_carryout=off  en=off  latency=0  precision=User Defined  arith_type="Signed  (2's comp)"  n_bits=22  bin_pt=16  quantization=Truncate  overflow=Flag as error  dbl_ovrd=off  use_behavioral_HDL=on  pipelined=off  use_rpm=on  hw_selection=Fabric  block_type=addsub
      settings: xl_area=[9 0 0 16 0 0 0]  block_version=VER_STRING_GOES_HERE
  Add2 : xbsIndex_r4/AddSub  mode=Subtraction  use_carryin=off  use_carryout=off  en=off  latency=0  precision=User Defined  arith_type="Signed  (2's comp)"  n_bits=22  bin_pt=16  quantization=Truncate  overflow=Flag as error  dbl_ovrd=off  use_behavioral_HDL=on  pipelined=off  use_rpm=on  hw_selection=Fabric  block_type=addsub
      settings: xl_area=[9 0 0 16 0 0 0]  block_version=VER_STRING_GOES_HERE
  ComplexMult_x1 : Subsystem
  ComplexMult_x2 : Subsystem
  Re : Outport  OutDataType=sfix(16)  OutScaling=2^0
  Im : Outport  Port=2  OutDataType=sfix(16)  OutScaling=2^0
  wires:
  ComplexMult_x2/1(Re) -> Add2/1
  ComplexMult_x1/1(Re) -> Add2/2
  ComplexMult_x2/2(Im) -> Add1/1
  ComplexMult_x1/2(Im) -> Add1/2
  Re[a]/1 -> ComplexMult_x2/1(Re[A])
  Im[a]/1 -> ComplexMult_x2/2(Im[A])
  Add2/1 -> Re/1
  Re[d]/1 -> ComplexMult_x2/3(Re[B])
  Im[d]/1 -> ComplexMult_x2/4(Im[B])
  Re[b]/1 -> ComplexMult_x1/1(Re[A])
  Add1/1 -> Im/1
  Im[b]/1 -> ComplexMult_x1/2(Im[A])
  Re[c]/1 -> ComplexMult_x1/3(Re[B])
  Im[c]/1 -> ComplexMult_x1/4(Im[B])
